simulink model "TosAeb"  (R2021a)
settings: Solver=FixedStepDiscrete  StartTime=0.0  StopTime=inf  FixedStep=0.01  RelTol=1e-3
undefined symbols (not defined in the file or in its folder): ACCModSts, AccSts1, AEB_ObjectMovingOrientation, AEB_ObjectMovingState, CN_BTCActive, CN_CurrentGearR, CN_CurrentGearR_DHT, CN_PTCActive, CN_VDCActive, CN_VSC_EspOff, CN_VSC_FCW_AEB_Error, CN_VSC_FCW_AEB_NoDispay, CN_VSC_FCW_AEB_PerfmDgdtn, CN_VSC_FCW_AEB_TempUnAvlbl, CN_VSC_VehStandstill, CNaccelAvoidMax, CNaccelAvoidMin, CNbrakePedalPress, CNcomfortStrLeftAccelMax, CNcomfortStrLeftAccelMin, CNcomfortStrRightAccelMax, CNcomfortStrRightAccelMin, CNDrvDoorOpen, CNDrvModeEco, CNDrvModeNorm, CNDrvModeOther, CNDrvModeSport, CNDrvSBRreminder, CNNull, CNobjInvalidID, CNPassDoorOpen, CNPassSBRreminder, CNPEBSAccelNegInf, CNPEBSAccelNull, CNPEBSTimeInf, CNPEBSTimeNull, CNSTOPMAX, CNSTOPNULL, CNsubjWidth, CNSwtOFF, CNSwtON, CNTTCMAX, CNTTCNULL, CNVehConfigAEBCycNone, CNVehConfigAEBNone, CNVehConfigAEBPedNone, CNVehConfigFCWNone, CNVehConfigJANone, CollisionSeverity, FALSE, FCWSnvty, JACycObjCalcInfoDefaultParams, JAPedObjCalcInfoDefaultParams, JAVehObjCalcInfoDefaultParams, latCycObjCalcInfoDefaultParams, latPedObjCalcInfoDefaultParams, longObjCalcInfoDefaultParams, ModSts, movingStatus, ObjectType ... (+1112)
libraries: simulink x3031, AEBSFunctionLib x50, Ta_Library x33, AEBSStateMachineLib x23, Mylibrary x12, simulink_extras x7, ObjSelect_lib x3, dspstat3 x2
(part 102 of 102)

== stateflow chart TosAeb/FcwAebJa_Step_sys/ALG_AEBSystem/ALG_AEBController/AEB_FuncImpManagementApp/FuncManeuArbCalc/brkFuncManeuArbCalc/axTgtReqValueCalc1/Fctb_IsNeedBrakeCalc/IsNeedBrakeFlow ==
  state Distance = Distance_Cal1(subjVel,DistanceK1) [function]
  state Distance = Distance_Cal2(subjVel,Fctb_AxReq) [function]
  state StopState
    en,du:
    FctbState = uint8(0);
    Distance = 0;
    Distance1 = 0;
    Distance2 = 0;
    IsNeedBrake = false;
  state IsNeedBrakeCal [function]
    (default) -> (junction 34)
    (junction 34) -> (junction 36) [order 1] : [FctbReq == true &&...
        | Distance < P_AEB_FIM_FctbDistThes]
    (junction 34) -> (junction 40) [order 2] : {
        |    IsNeedBrakeCond = false;
        | }
    (junction 36) -> (junction 38) : {
        |    IsNeedBrakeCond = true;
        | }
    (junction 38) -> (junction 40)
    (junction 40) -> (junction 43)
  state DriveOff
    en:
    FctbState = uint8(1);
    IsNeedBrake = false;
    Distance1 = 0;
    Distance = 0;
    du:
    IsNeedBrake = false;
    Distance1 = Distance_Cal1(subjVel,Distance1);
    Distance = Distance1;
  state DriveOn
    en:IsNeedBrake = false;
    FctbState = uint8(4);
    Distance = 0;
    Distance1 = 0;
    Distance2 = 0;
  state BrakeState
    en,du: Distance2 = Distance_Cal2(subjVel,Fctb_AxReq);
    Distance = Distance1+Distance2;
    IsNeedBrakeCal();
    state NoNeedBrake
      en,du:
      FctbState = uint8(2);
      IsNeedBrake = false;
    state IsNeedBrake
      en,du:
      FctbState = uint8(3);
      IsNeedBrake = true;
    (default) -> (junction 76)
    (junction 76) -> NoNeedBrake [order 2]
    (junction 76) -> IsNeedBrake [order 1] : [IsNeedBrakeCond == true]
    NoNeedBrake -> IsNeedBrake : [IsNeedBrakeCond == true]
  (default) -> (junction 12)
  (junction 12) -> (junction 13) [order 2]
  (junction 12) -> StopState [order 1] : [standstill == true]
  (junction 13) -> DriveOn
  (junction 49) -> StopState
  (junction 59) -> StopState : [standstill == true&&...
      | FctbReq == false]
  (junction 47) -> (junction 49)
  DriveOn -> (junction 59)
  DriveOff -> StopState [order 3] : [standstill == true]
  StopState -> DriveOff : [standstill == false]
  DriveOff -> DriveOn [order 2] : [standstill == false&&...
      | Distance >=P_AEB_FIM_FctbDistThes]
  (junction 54) -> DriveOn
  DriveOff -> BrakeState [order 1] : [FctbReq == true &&...
      | Distance < P_AEB_FIM_FctbDistThes]
  BrakeState -> (junction 47) [order 1] : [standstill == true&&...
      | FctbReq == false]
  BrakeState -> (junction 54) [order 2] : [standstill == false&&...
      | FctbReq == false]
  data: subjVel function_input; Distance function_output; DistanceK1 function_input; subjVel function_input; Fctb_AxReq function_input; Distance function_output; IsNeedBrake output boolean; FctbState output uint8; Distance output single; FctbReq input boolean; standstill input uint8; subjVel input single; Fctb_AxReq input single; P_AEB_FIM_FctbDistThes input single; Distance1 output single; Distance2 output single; IsNeedBrakeCond local boolean

== stateflow chart TosAeb/FcwAebJa_Step_sys/ALG_AEBSystem/ALG_AEBOutput/AEBS_IPHUTInfoCalc/AR_HUD_InfoMap/AEB_RiPedFlow ==
  state TargetSelectFunc [function]
    (default) -> (junction 63)
    (junction 63) -> (junction 75) [order 1] : [VehicleConfigAEBPed == true &&...
        | latPedCalcInfo(index).latPedObjRawInfo.ObjectID ~= CNobjInvalidID &&...
        | latPedCalcInfo(index).latPedObjRawInfo.ObjectType == ObjectTypeCopy.PEDESTRIAN &&...
        | latPedCalcInfo(index).latPedObjRawInfo.dy < 0 && ...
        | latPedCalcInfo(index).latPedObjRawInfo.dx > 0 &&...
        | latPedCalcInfo(index).CollisionRiskProb > 0.2]
    (junction 63) -> (junction 230) [order 2]
    (junction 75) -> (junction 213) : {
        | 	tgtIsFind = true;
        | }
    (junction 213) -> (junction 215) [order 1] : [(VehicleConfigAEBPed == true &&...
        | latPedCalcInfo(tgtIndex).latPedObjRawInfo.ObjectID ~= CNobjInvalidID &&...
        | latPedCalcInfo(tgtIndex).latPedObjRawInfo.ObjectType == ObjectTypeCopy.PEDESTRIAN &&...
        | latPedCalcInfo(tgtIndex).latPedObjRawInfo.dy < 0 && ...
        | latPedCalcInfo(tgtIndex).latPedObjRawInfo.dx > 0 &&...
        | latPedCalcInfo(index).CollisionRiskProb > 0.2) == false]
    (junction 213) -> (junction 128) [order 2] : {
        | 	temp1= latPedCalcInfo(index).latPedObjRawInfo.dx;
        | 	temp2 = latPedCalcInfo(tgtIndex).latPedObjRawInfo.dx;
        | }
    (junction 215) -> (junction 218) : {
        | 	tgtIndex = index;
        | }
    (junction 128) -> (junction 131) [order 1] : [temp1 < temp2]
    (junction 128) -> (junction 101) [order 2]
    (junction 131) -> (junction 133) : {
        | 	tgtIndex = index;
        | }
    (junction 101) -> (junction 230)
    (junction 133) -> (junction 101)
    (junction 218) -> (junction 133)
    (junction 230) -> (junction 233)
  note: AEB_LePedDetn
      |
      | AEB_LePedTyp
      |
      | AEB_LePedHeading
      |
      | AEB_LePedDx
      |
      | AEB_LePedDy
      |
      | AEB_LePed_collisionprob
  (default) -> (junction 112) : {
      | 	tgtIndex = 1;
      | 	tgtIsFind = false;
      |       index = uint8(1);
      | }
  (junction 112) -> (junction 110) [order 1] : [index <= 10]
  (junction 169) -> (junction 112)
  (junction 110) -> (junction 113) : {
      | 	TargetSelectFunc();
      | }
  (junction 112) -> (junction 114) [order 2]
  (junction 113) -> (junction 169) : {
      | 	index = index + 1;
      | }
  (junction 114) -> (junction 172) [order 1] : [tgtIsFind == true]
  (junction 114) -> (junction 176) [order 2] : {
      | 	tgtObj = latPedObjCalcInfoDefaultParams;
      | }
  (junction 172) -> (junction 174) : {
      | 	tgtObj = latPedCalcInfo(tgtIndex);
      | }
  (junction 174) -> (junction 176)
  (junction 176) -> (junction 179)
  (junction 179) -> (junction 235) [order 1] : [tgtObj.latPedObjRawInfo.ObjectID ~= CNobjInvalidID]
  (junction 179) -> (junction 240) [order 2] : {
      |   AEB_RiPedDetn = false;
      |   AEB_RiPedTyp = uint8(0);
      |   AEB_RiPedHeading = single(0);
      |   AEB_RiPedDx = single(0);
      |   AEB_RiPedDy = single(0);
      |   AEB_RiPed_collisionprob = false;
      | }
  (junction 235) -> (junction 237) : {
      |   AEB_RiPedDetn = true;
      |   AEB_RiPedTyp = uint8(4);
      |   AEB_RiPedHeading = tgtObj.latPedObjRawInfo.ObjectAzimuthAngle;
      |   AEB_RiPedDx = max((tgtObj.latPedObjRawInfo.dx - P_Global_WheelBase_m),0);
      |   AEB_RiPedDy = -tgtObj.latPedObjRawInfo.dy;
      |   AEB_RiPed_collisionprob = AEB_Ped_collisionprob;
      | }
  (junction 237) -> (junction 240)
  (junction 240) -> (junction 243)
  data: AEB_Ped_collisionprob input; VehicleConfigAEBPed input; latPedCalcInfo input Bus: BusLatPedObjCalcInfo size=10; index local uint8; temp1 local; temp2 local; latPedObjCalcInfoDefaultParams parameter Bus: BusLatPedObjCalcInfo; CNobjInvalidID parameter; AEB_RiPedDetn output; AEB_RiPedTyp output; AEB_RiPedHeading output; AEB_RiPedDx output; AEB_RiPedDy output; AEB_RiPed_collisionprob output; tgtIsFind local; tgtObj local Bus: BusLatPedObjCalcInfo; tgtIndex local uint8; P_Global_WheelBase_m parameter

== stateflow chart TosAeb/FcwAebJa_Step_sys/ALG_AEBSystem/ALG_AEBOutput/AEBS_IPHUTInfoCalc/FCW_AEB_FctStsCalc/FCW_AEB_FctStsCalc ==
  note: 0x00:No display
      | 0x01:error
      | 0x02:FCW/AEB_V temporarily unavailable
      | 0x03:FCW/AEB_V Performance degradation
  (default) -> (junction 80)
  (junction 80) -> (junction 82) [order 1] : [AEB_VehResp == TRUE||...
      | AEB_PedResp == TRUE ||...
      | AEB_JAResp == TRUE]
  (junction 80) -> (junction 98) [order 2] : {
      | 	FCW_AEB_VehFctSts = ...
      | CN_VSC_FCW_AEB_NoDispay;
      | }
  (junction 82) -> (junction 187)
  (junction 187) -> (junction 189) [order 1] : [FCW_AEB_ErrorState == TRUE]
  (junction 187) -> (junction 191) [order 2]
  (junction 189) -> (junction 5) : {
      | 	FCW_AEB_VehFctSts = ...
      | CN_VSC_FCW_AEB_Error;
      | }
  (junction 191) -> (junction 193) [order 1] : [EspFuncOffSts == CN_VSC_EspOff]
  (junction 191) -> (junction 195) [order 2]
  (junction 193) -> (junction 88) : {
      | 	FCW_AEB_VehFctSts = ...
      | CN_VSC_FCW_AEB_PerfmDgdtn;
      | }
  (junction 195) -> (junction 86) [order 1] : [TrailerSts == TRUE]
  (junction 195) -> (junction 197) [order 2] : {
      | 	FCW_AEB_VehFctSts = ...
      | CN_VSC_FCW_AEB_NoDispay;
      | }
  (junction 86) -> (junction 94) : {
      | 	FCW_AEB_VehFctSts = ...
      | CN_VSC_FCW_AEB_TempUnAvlbl;
      | }
  (junction 197) -> (junction 98)
  (junction 94) -> (junction 197)
  (junction 88) -> (junction 94)
  (junction 5) -> (junction 88)
  (junction 98) -> (junction 103)
  (junction 103) -> (junction 156) [order 1] : [AEB_PedResp == TRUE&&...
      | AEB_PEDP_ErrorState == TRUE]
  (junction 103) -> (junction 160) [order 2] : {
      | 	FCW_AEB_PedFctSts = ...
      | CN_VSC_FCW_AEB_NoDispay;
      | }
  (junction 156) -> (junction 158) : {
      | 	FCW_AEB_PedFctSts = ...
      | CN_VSC_FCW_AEB_TempUnAvlbl;
      | }
  (junction 158) -> (junction 160)
  (junction 160) -> (junction 174)
  (junction 174) -> (junction 179) [order 1] : [AEB_JAResp == TRUE&&...
      | AEB_JA_ErrorState == TRUE]
  (junction 174) -> (junction 176) [order 2] : {
      | 	AEB_JAFuncSts = ...
      | CN_VSC_FCW_AEB_NoDispay;
      | }
  (junction 179) -> (junction 180) : {
      | 	AEB_JAFuncSts = ...
      | CN_VSC_FCW_AEB_TempUnAvlbl;
      | }
  (junction 180) -> (junction 176)
  (junction 176) -> (junction 183)
  data: FCW_AEB_VehFctSts output uint8; AEB_VehResp input; AEB_PedResp input; AEB_JAResp input; FCW_AEB_PedFctSts output uint8; FCW_AEB_ErrorState input; AEB_PEDP_ErrorState input; AEB_JA_ErrorState input; CN_VSC_FCW_AEB_TempUnAvlbl parameter; EspFuncOffSts input; TrailerSts input; TRUE parameter; CN_VSC_FCW_AEB_PerfmDgdtn parameter; CN_VSC_FCW_AEB_NoDispay parameter; CN_VSC_FCW_AEB_Error parameter; CN_VSC_EspOff parameter; AEB_JAFuncSts output uint8
